simulink model "PrkgClimaActvMgr"  (R2022b) (continued)
== system PrkgClimaActvMgr/PrkgClimaActvMgr_Runnable_sys == (continued)
      settings: EnsureOutportIsVirtual=on
  PrkgClimaOnReq_PrkgClimaOnReq_write : Outport  Port=116
      settings: EnsureOutportIsVirtual=on
  PrkgClimaPostCdngReq_PrkgClimaPostCdngReq_write : Outport  Port=117
      settings: EnsureOutportIsVirtual=on
  PrkgClimaPreCdngReq_PrkgClimaPreCdngReq_write : Outport  Port=118
      settings: EnsureOutportIsVirtual=on
  PrkgClimaRecirReq_PrkgClimaRecirReq_write : Outport  Port=119
      settings: EnsureOutportIsVirtual=on
  PrkgClimaRqrdImdtForBattULo_PrkgClimaRqrdImdtForBattULo_write : Outport  Port=120
      settings: EnsureOutportIsVirtual=on
  PrkgClimaRqrdImdtFromActvMgr_PrkgClimaRqrdImdtFromActvMgr_write : Outport  Port=121
      settings: EnsureOutportIsVirtual=on
  PrkgClimaRqrdTmrForBattULo_PrkgClimaRqrdTmrForBattULo_write : Outport  Port=122
      settings: EnsureOutportIsVirtual=on
  PrkgClimaTemp_PrkgClimaTemp_write : Outport  Port=123
      settings: EnsureOutportIsVirtual=on
  PrkgClimaWarn_PrkgClimaWarn_write : Outport  Port=124
      settings: EnsureOutportIsVirtual=on
  PrkgModMgr_PrkgModMgr_write : Outport  Port=125
      settings: EnsureOutportIsVirtual=on
  RemClimaActv_RemClimaActv_write : Outport  Port=126
      settings: EnsureOutportIsVirtual=on
  RemClimaDefrstSts_RemClimaDefrstSts_write : Outport  Port=127
      settings: EnsureOutportIsVirtual=on
  RemClimaDelaySts_RemClimaDelaySts_write : Outport  Port=128
      settings: EnsureOutportIsVirtual=on
  RemClimaEgyFinalReq_RemClimaEgyFinalReq_write : Outport  Port=129
      settings: EnsureOutportIsVirtual=on
  RemClimaExtnTiRspn_RemClimaExtnTiRspn_write : Outport  Port=130
      settings: EnsureOutportIsVirtual=on
  RemClimaFnshd_RemClimaFnshd_write : Outport  Port=131
      settings: EnsureOutportIsVirtual=on
  RemClimaHvSts_RemClimaHvSts_write : Outport  Port=132
      settings: EnsureOutportIsVirtual=on
  RemStrtClimaRspn_RemStrtClimaRspn_write : Outport  Port=133
      settings: EnsureOutportIsVirtual=on
  RemVentActvSts_RemVentActvSts_write : Outport  Port=134
      settings: EnsureOutportIsVirtual=on
  RemVentFbSts_RemVentFbSts_write : Outport  Port=135
      settings: EnsureOutportIsVirtual=on
  RemVentReqRspnFb_RemVentReqRspnFb_write : Outport  Port=136
      settings: EnsureOutportIsVirtual=on
  RemVentWarnSts_RemVentWarnSts_write : Outport  Port=137
      settings: EnsureOutportIsVirtual=on
  ReqForPrkgClima_ReqForPrkgClima_write : Outport  Port=138
      settings: EnsureOutportIsVirtual=on
  ReqForSwtHeatrTypFromActvMgr_ReqForSwtHeatrTypFromActvMgr_write : Outport  Port=139
      settings: EnsureOutportIsVirtual=on
  RoofOpnReqFrmClima_RoofOpnReqFrmClima_write : Outport  Port=140
      settings: EnsureOutportIsVirtual=on
  RtLeForPrkgClima_RtLeForPrkgClima_write : Outport  Port=141
      settings: EnsureOutportIsVirtual=on
  SchedCmptHeatCoolFB_SchedCmptHeatCoolFB_write : Outport  Port=142
      settings: EnsureOutportIsVirtual=on
  SeatClimaDurgClima_SeatClimaDurgClima_write : Outport  Port=143
      settings: EnsureOutportIsVirtual=on
  SteerWhlHeatgDurgClima_Ngcc_SteerWhlHeatgDurgClima_write : Outport  Port=144
      settings: EnsureOutportIsVirtual=on
  SuspStrtActvForPrkgClima_SuspStrtActvForPrkgClima_write : Outport  Port=145
      settings: EnsureOutportIsVirtual=on
  WarnForHybWoUFromActvMgr_WarnForHybWoUFromActvMgr_write : Outport  Port=146
      settings: EnsureOutportIsVirtual=on
  wires:
  ActvRqrdForPostPrkgClima_ActvRqrdForPostPrkgClima_read/1 -> Terminator_1/1
  CarTiIntForClima_CarTiIntForClima_read/1 -> Terminator_2/1
  CdsClimaOnReqfromExsrc_CdsClimaOnReqfromExsrc_read/1 -> Terminator_3/1
  ClimaCfgForClimaCtrlMan1_ClimaCfgForClimaCtrlMan1_read/1 -> Terminator_4/1
  ClimaCfgForCmptmtClng_ClimaCfgForCmptmtClng_read/1 -> Terminator_5/1
  ClimaCfgForFoldCSD_ClimaCfgForFoldCSD_read/1 -> Terminator_6/1
  ClimaCfgForNrOfTSetg1_ClimaCfgForNrOfTSetg1_read/1 -> Terminator_7/1
  ClimaCfgForPreClng1_ClimaCfgForPreClng1_read/1 -> Terminator_8/1
  ClimaCfgForSeatHeatgForRowFirstPrsnt1_ClimaCfgForSeatHeatgForRowFirstPrsnt1_read/1 -> Terminator_9/1
  ClimaCfgForSeatHeatgForRowSec_ClimaCfgForSeatHeatgForRowSec_read/1 -> Terminator_10/1
  ClimaCfgForSeatVentnForRowFirst_ClimaCfgForSeatVentnForRowFirst_read/1 -> Terminator_11/1
  ClimaCfgForSeatVentnForRowSec_ClimaCfgForSeatVentnForRowSec_read/1 -> Terminator_12/1
  ClimaCfgForSteerWhlHeatgPrsnt1_ClimaCfgForSteerWhlHeatgPrsnt1_read/1 -> Terminator_13/1
  ClimaCfgForSteerWhlLeSide1_ClimaCfgForSteerWhlLeSide1_read/1 -> Terminator_14/1
  ClimaCfgForVehElec1_ClimaCfgForVehElec1_read/1 -> Terminator_15/1
  ClimaCfgForVehHyb1_ClimaCfgForVehHyb1_read/1 -> Terminator_16/1
  ClimaCfgForWindHeatrPrsnt1_ClimaCfgForWindHeatrPrsnt1_read/1 -> Terminator_17/1
  ClimaOvrHeatProWarnIni_ClimaOvrHeatProWarnIni_read/1 -> Terminator_18/1
  ClimaOvrHeatReqFromExrsc_ClimaOvrHeatReqFromExrsc_read/1 -> Terminator_19/1
  ClimaOvrHeatStrtReq_ClimaOvrHeatStrtReq_read/1 -> Terminator_20/1
  CmptmtClsd_CmptmtClsd_read/1 -> Terminator_21/1
  CSDUsgChgeOffReq_CSDUsgChgeOffReq_read/1 -> Terminator_22/1
  CSDUsgChgeOnReq_CSDUsgChgeOnReq_read/1 -> Terminator_23/1
  DefrstHeatgForPrkgClimaEnad_DefrstHeatgForPrkgClimaEnad_read/1 -> Terminator_24/1
  DoorOpenStsForClima_DoorOpenStsForClima_read/1 -> Terminator_25/1
  EgyAllwdForPrkgClima_EgyAllwdForPrkgClima_read/1 -> Terminator_26/1
  EgyPrioForPrkgClima_EgyPrioForPrkgClima_read/1 -> Terminator_27/1
  ErsCmdByKeyStrtReq_ErsCmdByKeyStrtReq_read/1 -> Terminator_28/1
  ErsRunTimelStrtReq_ErsRunTimelStrtReq_read/1 -> Terminator_29/1
  FacForRtForPrkgClima_FacForRtForPrkgClima_read/1 -> Terminator_30/1
  FrntHvacBlowerSts_FrntHvacBlowerSts_read/1 -> Terminator_31/1
  HeatrTypForPrkgClima_HeatrTypForPrkgClima_read/1 -> Terminator_32/1
  HeatUpRqrdForPrkgClima_HeatUpRqrdForPrkgClima_read/1 -> Terminator_33/1
  HMIExtVentFanVolReqStrtReq_HMIExtVentFanVolReqStrtReq_read/1 -> Terminator_34/1
  HMIExtVentonoffReqStrtReq_HMIExtVentonoffReqStrtReq_read/1 -> Terminator_35/1
  HMIExtVentTSpReqStrtReq_HMIExtVentTSpReqStrtReq_read/1 -> Terminator_36/1
  HMIExtVentTStsReqStrtReq_HMIExtVentTStsReqStrtReq_read/1 -> Terminator_37/1
  HvBattForPrkgClima_HvBattForPrkgClima_read/1 -> Terminator_38/1
  HvBattSoc_HvBattSoc_read/1 -> Terminator_39/1
  HvSysCnctWoU_HvSysCnctWoU_read/1 -> Terminator_40/1
  HvSysWoU_HvSysWoU_read/1 -> Terminator_41/1
  HvSysRlySts_HvSysRlySts_read/1 -> Terminator_42/1
  IntCo2MontrStrtReq_IntCo2MontrStrtReq_read/1 -> Terminator_43/1
  LockgCenSts_Ngcc_LockgCenSts_read/1 -> Terminator_44/1
  MemReadForPrkgClimaActvMgr_MemReadForPrkgClimaActvMgr_read/1 -> Terminator_45/1
  MemReqStsForPrkgClima_MemReqStsForPrkgClima_read/1 -> Terminator_46/1
  OffForTLoForPostCdng_OffForTLoForPostCdng_read/1 -> Terminator_47/1
  OffRqrdForPrkgClima_OffRqrdForPrkgClima_read/1 -> Terminator_48/1
  PrePrkgClimaNotAvlForHvSysWoU_PrePrkgClimaNotAvlForHvSysWoU_read/1 -> Terminator_49/1
  PrkgCDSAvl_PrkgCDSAvl_read/1 -> Terminator_50/1
  PrkgClimaAllwdForBattULo_PrkgClimaAllwdForBattULo_read/1 -> Terminator_51/1
  PrkgClimaAllwdForSwtHeatrTyp_PrkgClimaAllwdForSwtHeatrTyp_read/1 -> Terminator_52/1
  PrkgHeatgActv_PrkgHeatgActv_read/1 -> Terminator_53/1
  PwrAllwdForHvacFanFrnt_PwrAllwdForHvacFanFrnt_read/1 -> Terminator_54/1
  PwrCnsDesForHvacFanFrnt_PwrCnsDesForHvacFanFrnt_read/1 -> Terminator_55/1
  RemClimaallExsrcReq_RemClimaallExsrcReq_read/1 -> Terminator_56/1
  RemClimaEgyExsrcReq_RemClimaEgyExsrcReq_read/1 -> Terminator_57/1
  RemClimaTmrStrtReq_RemClimaTmrStrtReq_read/1 -> Terminator_58/1
  RemStrtExtnTiStrtReq_RemStrtExtnTiStrtReq_read/1 -> Terminator_59/1
  RemStrtHvCtrlStrtReq_RemStrtHvCtrlStrtReq_read/1 -> Terminator_60/1
  RemVentReqStrtReq_RemVentReqStrtReq_read/1 -> Terminator_61/1
  RtCmftForImdtPrePrkgClima_RtCmftForImdtPrePrkgClima_read/1 -> Terminator_62/1
  RtCmftForPostPrkgClima_RtCmftForPostPrkgClima_read/1 -> Terminator_63/1
  RtCmftForPreClng_RtCmftForPreClng_read/1 -> Terminator_64/1
  RtSdlForPrkgClima_RtSdlForPrkgClima_read/1 -> Terminator_65/1
  SeatHeatForPrkgClimaEnad_SeatHeatForPrkgClimaEnad_read/1 -> Terminator_66/1
  SteerWhlHeatgForPrkgClimaEnad_SteerWhlHeatgForPrkgClimaEnad_read/1 -> Terminator_67/1
  StrtRemSts1WdStrtRemSts_Ngcc_StrtRemSts1WdStrtRemSts_read/1 -> Terminator_68/1
  StrtRqrdForDirForPrePrkgClima_StrtRqrdForDirForPrePrkgClima_read/1 -> Terminator_69/1
  StrtRqrdForPreClng_StrtRqrdForPreClng_read/1 -> Terminator_70/1
  StrtRqrdForTmrForPrePrkgClima_StrtRqrdForTmrForPrePrkgClima_read/1 -> Terminator_71/1
  SuspStrtAllwdForPrkgClima_SuspStrtAllwdForPrkgClima_read/1 -> Terminator_72/1
  TelmBookClimaReqHmiCmptmtTSpSpclStrtReq_TelmBookClimaReqHmiCmptmtTSpSpclStrtReq_read/1 -> Terminator_73/1
  TelmBookClimaReqOnOffNoReqStrtReq_TelmBookClimaReqOnOffNoReqStrtReq_read/1 -> Terminator_74/1
  TelmBookClimaReqTempRangeStrtReq_TelmBookClimaReqTempRangeStrtReq_read/1 -> Terminator_75/1
  TelmBookClimaTmrStrtReq_TelmBookClimaTmrStrtReq_read/1 -> Terminator_76/1
  TelmBookSeatHeatClimalReqDrvHeatClimaLvlStrtReq_TelmBookSeatHeatClimalReqDrvHeatClimaLvlStrtReq_read/1 -> Terminator_77/1
  TelmBookSeatHeatClimalReqPassHeatClimaLvlStrtReq_TelmBookSeatHeatClimalReqPassHeatClimaLvlStrtReq_read/1 -> Terminator_78/1
  TelmBookSeatHeatClimalReqSecLeHeatClimaLvlStrtReq_TelmBookSeatHeatClimalReqSecLeHeatClimaLvlStrtReq_read/1 -> Terminator_79/1
  TelmBookSeatHeatClimalReqSecRiHeatClimaLvlStrtReq_TelmBookSeatHeatClimalReqSecRiHeatClimaLvlStrtReq_read/1 -> Terminator_80/1
  TelmBookSeatHeatClimalReqThrdLeHeatClimaLvlStrtReq_TelmBookSeatHeatClimalReqThrdLeHeatClimaLvlStrtReq_read/1 -> Terminator_81/1
  TelmBookSeatHeatClimalReqThrdRiHeatClimaLvlStrtReq_TelmBookSeatHeatClimalReqThrdRiHeatClimaLvlStrtReq_read/1 -> Terminator_82/1
  TelmBookSeatVentnClimalReqDrvVentnClimaLvlStrtReq_TelmBookSeatVentnClimalReqDrvVentnClimaLvlStrtReq_read/1 -> Terminator_83/1
  TelmBookSeatVentnClimalReqPassVentnClimaLvlStrtReq_TelmBookSeatVentnClimalReqPassVentnClimaLvlStrtReq_read/1 -> Terminator_84/1
  TelmBookSeatVentnClimalReqSecLeVentnClimaLvlStrtReq_TelmBookSeatVentnClimalReqSecLeVentnClimaLvlStrtReq_read/1 -> Terminator_85/1
  TelmBookSeatVentnClimalReqSecRiVentnClimaLvlStrtReq_TelmBookSeatVentnClimalReqSecRiVentnClimaLvlStrtReq_read/1 -> Terminator_86/1
  TelmBookSeatVentnClimalReqThrdLeVentnClimaLvlStrtReq_TelmBookSeatVentnClimalReqThrdLeVentnClimaLvlStrtReq_read/1 -> Terminator_87/1
  TelmBookSeatVentnClimalReqThrdRiVentnClimaLvlStrtReq_TelmBookSeatVentnClimalReqThrdRiVentnClimaLvlStrtReq_read/1 -> Terminator_88/1
  TelmBookSteerWhlHeatClimalReqStrtReq_TelmBookSteerWhlHeatClimalReqStrtReq_read/1 -> Terminator_89/1
  TelmClimaExtVentnLvlStrtReq_TelmClimaExtVentnLvlStrtReq_read/1 -> Terminator_90/1
  TelmClimaExtVentnReqStrtReq_TelmClimaExtVentnReqStrtReq_read/1 -> Terminator_91/1
  TelmClimaExtVentnTempRangeStrtReq_TelmClimaExtVentnTempRangeStrtReq_read/1 -> Terminator_92/1
  TelmClimaExtVentnTSpSpclStrtReq_TelmClimaExtVentnTSpSpclStrtReq_read/1 -> Terminator_93/1
  UpdRtTotForTmrForPrePrkgClima_UpdRtTotForTmrForPrePrkgClima_read/1 -> Terminator_94/1
  VehModMngtGlbSafe1_Ngcc_VehModMngtGlbSafe1_read/1 -> Terminator_95/1
  VFCBodyPreClimatisationHmiHumCtrl_VFCBodyPreClimatisationHmiHumCtrl_read/1 -> Terminator_96/1
  VFCHmiClimaCtrlAct_VFCHmiClimaCtrlAct_read/1 -> Terminator_97/1
  VFCPrkDrvgClimabyFragAct_VFCPrkDrvgClimabyFragAct_read/1 -> Terminator_98/1
  WarnForEgyAllwdLimd_WarnForEgyAllwdLimd_read/1 -> Terminator_99/1
  WarnForHybWoU_WarnForHybWoU_read/1 -> Terminator_100/1
  WarnForPreCdngFctNotAvl_WarnForPreCdngFctNotAvl_read/1 -> Terminator_101/1
  WarnForPrkgClimaNotAllwd_WarnForPrkgClimaNotAllwd_read/1 -> Terminator_102/1
  WindIceDetdFrnt_Ngcc_WindIceDetdFrnt_read/1 -> Terminator_103/1
  WindIceDetdRe_Ngcc_WindIceDetdRe_read/1 -> Terminator_104/1
  AirCdnAvl_AirCdnAvl_read/1 -> Terminator_105/1
  AmbTFildForClimaNgcc_AmbTFildForClima_read/1 -> Terminator_106/1
  OffRqrdForRemClima_OffRqrdForRemClima_read/1 -> Terminator_107/1
  RemClimaTempStrtReq_RemClimaTempStrtReq_read/1 -> Terminator_108/1
  VFCAutDemstOperSts_VFCAutDemstOperSts_read/1 -> Terminator_109/1
  VFCPrkDrvgClimabyPM25_VFCPrkDrvgClimabyPM25_read/1 -> Terminator_110/1
  AirCdnAvlRe_AirCdnAvlRe_read/1 -> Terminator_111/1
  AirSrcStsFbNgcc_AirSrcStsFbNgcc_read/1 -> Terminator_112/1
  BookTrvlClimaActvReqfromExsrc_BookTrvlClimaActvReqfromExsrc_read/1 -> Terminator_113/1
  CCMWakeupFunction_CCMWakeupFunction_read/1 -> Terminator_114/1
  ClimaCfgFor3RDROWSEATHEAT_ClimaCfgFor3RDROWSEATHEAT_read/1 -> Terminator_115/1
  ClimaCfgFor3RDROWSEATVENTILATED_ClimaCfgFor3RDROWSEATVENTILATED_read/1 -> Terminator_116/1
  ClimaCfgForAirMtrlSnsrPrsnt1_ClimaCfgForAirMtrlSnsrPrsnt1_read/1 -> Terminator_117/1
  ClimaCfgForBRAND_ClimaCfgForBRAND_read/1 -> Terminator_118/1
  ClimaCfgForDrvrSeatVentWthSmf2_ClimaCfgForDrvrSeatVentWthSmf2_read/1 -> Terminator_119/1
  ClimaCfgForErev1_ClimaCfgForErev1_read/1 -> Terminator_120/1
  ClimaCfgForHvacRePrsnt1_ClimaCfgForHvacRePrsnt1_read/1 -> Terminator_121/1
  ClimaCfgForHvacTyp_ClimaCfgForHvacTyp_read/1 -> Terminator_122/1
  ClimaCfgForLifeDetection_ClimaCfgForLifeDetection_read/1 -> Terminator_123/1
  ClimaCfgForMARKET_ClimaCfgForMARKET_read/1 -> Terminator_124/1
  ClimaCfgForPassSeatVentWthSmf2_ClimaCfgForPassSeatVentWthSmf2_read/1 -> Terminator_125/1
  ClimaCfgForPrpsnTyp_ClimaCfgForPrpsnTyp_read/1 -> Terminator_126/1
  ClimaCfgForRoofHatch_ClimaCfgForRoofHatch_read/1 -> Terminator_127/1
  ClimaCfgForVehTyp_ClimaCfgForVehTyp_read/1 -> Terminator_128/1
  ClimaCfgReqVersionReplace_ClimaCfgReqVersionReplace_read/1 -> Terminator_129/1
  CmptmtClngActv_CmptmtClngActv_read/1 -> Terminator_130/1
  CmptmtOpenStsForClima_CmptmtOpenStsForClima_read/1 -> Terminator_131/1
  DoorStsWithOutTrForClima_DoorStsWithOutTrForClima_read/1 -> Terminator_132/1
  DrvrSeatHeatgLvlSts_DrvrSeatHeatgLvlSts_read/1 -> Terminator_133/1
  DrvrSeatHeatgLvlSts_DrvrSeatHeatgLvlSts_IsUpdated_read/1 -> Terminator_134/1
  DrvrSeatVentnLvlSts_DrvrSeatVentnLvlSts_read/1 -> Terminator_135/1
  DrvrSeatVentnLvlSts_DrvrSeatVentnLvlSts_IsUpdated_read/1 -> Terminator_136/1
  DrvrSeatVentnLvlSts2Ngcc_DrvrSeatVentnLvlSts2Ngcc_read/1 -> Terminator_137/1
  EngSt1WdSts_Ngcc_EngSt1WdSts_read/1 -> Terminator_138/1
  ErsCmdByKeyFromExsrc_ErsCmdByKeyFromExsrc_read/1 -> Terminator_139/1
  GearLvrIndcn_GearLvrIndcn_read/1 -> Terminator_140/1
  GearLvrIndcn_GearLvrIndcn_IsUpdated_read/1 -> Terminator_141/1
  HighTempCleanHmiReqNgcc_HighTempCleanHmiReqNgcc_read/1 -> Terminator_142/1
  HMIAirCdnrAutDrySetNgcc_HMIAirCdnrAutDrySetNgcc_read/1 -> Terminator_143/1
  HMIAirCdnrAutDrySetNgcc_HMIAirCdnrAutDrySetNgcc_IsUpdated_read/1 -> Terminator_144/1
  HmiCmptmtTSp_HmiCmptmtTSp_read/1 -> Terminator_145/1
  HMILBRACStrtSetNgcc_HMILBRACStrtSetNgcc_read/1 -> Terminator_146/1
  HmiParkVentSetNgcc_HmiParkVentSetNgcc_read/1 -> Terminator_147/1
  HmiParkVentTmrSetNgcc_HmiParkVentTmrSetNgcc_read/1 -> Terminator_148/1
  HvBattMntnActv_HvBattMntnActv_read/1 -> Terminator_149/1
  HvCooltHeatrStsSig_HvCooltHeatrStsSig_read/1 -> Terminator_150/1
  HvCooltHeatrStsSig_HvCooltHeatrStsSig_IsUpdated_read/1 -> Terminator_151/1
  IntPm25VluFrmClima_IntPm25VluFrmClima_read/1 -> Terminator_152/1
  LDACState_LDACState_read/1 -> Terminator_153/1
  MemBookTrvlClimaTi_MemBookTrvlClimaTi_read/1 -> Terminator_154/1
  MemReadForBlwAftRun_MemReadForBlwAftRun_read/1 -> Terminator_155/1
  MemReadForHighTempCln_MemReadForHighTempCln_read/1 -> Terminator_156/1
  MemReadForHMISetEngReq_MemReadForHMISetEngReq_read/1 -> Terminator_157/1
  MemReadForParkVent_MemReadForParkVent_read/1 -> Terminator_158/1
  MemReadForParkVentActvCnt_MemReadForParkVentActvCnt_read/1 -> Terminator_159/1
  OnBdChrgrSt_OnBdChrgrSt_read/1 -> Terminator_160/1
  OnBdChrgrSt_OnBdChrgrSt_IsUpdated_read/1 -> Terminator_161/1
  OxygenSplyAvlStsNgcc_OxygenSplyAvlStsNgcc_read/1 -> Terminator_162/1
  OxygenSplyErrNgcc_OxygenSplyErrNgcc_read/1 -> Terminator_163/1
  PassSeatHeatgLvlSts_PassSeatHeatgLvlSts_read/1 -> Terminator_164/1
  PassSeatHeatgLvlSts_PassSeatHeatgLvlSts_IsUpdated_read/1 -> Terminator_165/1
  PassSeatVentnLvlSts_PassSeatVentnLvlSts_read/1 -> Terminator_166/1
  PassSeatVentnLvlSts_PassSeatVentnLvlSts_IsUpdated_read/1 -> Terminator_167/1
  PassSeatVentnLvlSts2Ngcc_PassSeatVentnLvlSts2Ngcc_read/1 -> Terminator_168/1
  PrkgClimaReqExcpSeat_PrkgClimaReqExcpSeat_read/1 -> Terminator_169/1
  PrkgClimaReqPrio_PrkgClimaReqPrio_read/1 -> Terminator_170/1
  PrkgOGMAirSrcReq_PrkgOGMAirSrcReq_read/1 -> Terminator_171/1
  PrvntWinActvReq_PrvntWinActvReq_read/1 -> Terminator_172/1
  ReHvacBlowerSts_ReHvacBlowerSts_read/1 -> Terminator_173/1
  RemClimaEgyExsrcReqPlus_RemClimaEgyExsrcReqPlus_read/1 -> Terminator_174/1
  RemClimaHVReqFb_RemClimaHVReqFb_read/1 -> Terminator_175/1
  RemClimaHVReqFb_RemClimaHVReqFb_IsUpdated_read/1 -> Terminator_176/1
  RemClimaWarnForCmptmtClng_RemClimaWarnForCmptmtClng_read/1 -> Terminator_177/1
  RemClimaWarnForOpenGlb_RemClimaWarnForOpenGlb_read/1 -> Terminator_178/1
  RemClimaWarnForUnLckClima_RemClimaWarnForUnLckClima_read/1 -> Terminator_179/1
  RemOxygenSplyReq_RemOxygenSplyReq_read/1 -> Terminator_180/1
  SeatHeatgLvlStsRowSecLe_SeatHeatgLvlStsRowSecLe_read/1 -> Terminator_181/1
  SeatHeatgLvlStsRowSecLe_SeatHeatgLvlStsRowSecLe_IsUpdated_read/1 -> Terminator_182/1
  SeatHeatgLvlStsRowSecRi_SeatHeatgLvlStsRowSecRi_read/1 -> Terminator_183/1
  SeatHeatgLvlStsRowSecRi_SeatHeatgLvlStsRowSecRi_IsUpdated_read/1 -> Terminator_184/1
  SeatHeatgLvlStsRowThrdLe_SeatHeatgLvlStsRowThrdLe_read/1 -> Terminator_185/1
  SeatHeatgLvlStsRowThrdLe_SeatHeatgLvlStsRowThrdLe_IsUpdated_read/1 -> Terminator_186/1
  SeatHeatgLvlStsRowThrdRi_SeatHeatgLvlStsRowThrdRi_read/1 -> Terminator_187/1
  SeatHeatgLvlStsRowThrdRi_SeatHeatgLvlStsRowThrdRi_IsUpdated_read/1 -> Terminator_188/1
  SeatVentnLvlStsRowSecLe_SeatVentnLvlStsRowSecLe_read/1 -> Terminator_189/1
  SeatVentnLvlStsRowSecLe_SeatVentnLvlStsRowSecLe_IsUpdated_read/1 -> Terminator_190/1
  SeatVentnLvlStsRowSecRi_SeatVentnLvlStsRowSecRi_read/1 -> Terminator_191/1
  SeatVentnLvlStsRowSecRi_SeatVentnLvlStsRowSecRi_IsUpdated_read/1 -> Terminator_192/1
  SeatVentnLvlStsRowThrdLe_SeatVentnLvlStsRowThrdLe_read/1 -> Terminator_193/1
  SeatVentnLvlStsRowThrdLe_SeatVentnLvlStsRowThrdLe_IsUpdated_read/1 -> Terminator_194/1
  SeatVentnLvlStsRowThrdRi_SeatVentnLvlStsRowThrdRi_read/1 -> Terminator_195/1
  SeatVentnLvlStsRowThrdRi_SeatVentnLvlStsRowThrdRi_IsUpdated_read/1 -> Terminator_196/1
  SteerWhlHeatgDurgClimaEnadFromExsrc_SteerWhlHeatgDurgClimaEnadFromExsrc_read/1 -> Terminator_197/1
  SunRoofPosnSts_Ngcc_SunRoofPosnSts_read/1 -> Terminator_198/1
  TcsOvrheatdProtnReq_TcsOvrheatdProtnReq_read/1 -> Terminator_199/1
  TelmClimaTmrFromExtSrc_TelmClimaTmrFromExtSrc_read/1 -> Terminator_200/1
  TelmDefrostTmr_TelmDefrostTmr_read/1 -> Terminator_201/1
  TelmDefrostTmr_TelmDefrostTmr_IsUpdated_read/1 -> Terminator_202/1
  TelmOxygenReqFromExSrc_TelmOxygenReqFromExSrc_read/1 -> Terminator_203/1
  TelmOxygenTmr_TelmOxygenTmr_read/1 -> Terminator_204/1
  TelmOxygenTmr_TelmOxygenTmr_IsUpdated_read/1 -> Terminator_205/1
  TelmSeatDrvrHeatReqFromExsrc_TelmSeatDrvrHeatReqFromExsrc_read/1 -> Terminator_206/1
  TelmSeatDrvVentnReqFromExsrc_TelmSeatDrvVentnReqFromExsrc_read/1 -> Terminator_207/1
  TelmSeatHeatDrvrTmr_TelmSeatHeatDrvrTmr_read/1 -> Terminator_208/1
  TelmSeatHeatDrvrTmr_TelmSeatHeatDrvrTmr_IsUpdated_read/1 -> Terminator_209/1
  TelmSeatHeatPassTmr_TelmSeatHeatPassTmr_read/1 -> Terminator_210/1
  TelmSeatHeatPassTmr_TelmSeatHeatPassTmr_IsUpdated_read/1 -> Terminator_211/1
  TelmSeatHeatSecLeTmr_TelmSeatHeatSecLeTmr_read/1 -> Terminator_212/1
  TelmSeatHeatSecLeTmr_TelmSeatHeatSecLeTmr_IsUpdated_read/1 -> Terminator_213/1
  TelmSeatHeatSecRiTmr_TelmSeatHeatSecRiTmr_read/1 -> Terminator_214/1
  TelmSeatHeatSecRiTmr_TelmSeatHeatSecRiTmr_IsUpdated_read/1 -> Terminator_215/1
  TelmSeatHeatThrdLeTmr_TelmSeatHeatThrdLeTmr_read/1 -> Terminator_216/1
  TelmSeatHeatThrdLeTmr_TelmSeatHeatThrdLeTmr_IsUpdated_read/1 -> Terminator_217/1
  TelmSeatHeatThrdRiTmr_TelmSeatHeatThrdRiTmr_read/1 -> Terminator_218/1
  TelmSeatHeatThrdRiTmr_TelmSeatHeatThrdRiTmr_IsUpdated_read/1 -> Terminator_219/1
  TelmSeatPassHeatReqFromExsrc_TelmSeatPassHeatReqFromExsrc_read/1 -> Terminator_220/1
  TelmSeatPassVentnReqFromExsrc_TelmSeatPassVentnReqFromExsrc_read/1 -> Terminator_221/1
  TelmSeatSecLeHeatReqFromExsrc_TelmSeatSecLeHeatReqFromExsrc_read/1 -> Terminator_222/1
  TelmSeatSecLeVentnReqFromExsrc_TelmSeatSecLeVentnReqFromExsrc_read/1 -> Terminator_223/1
  TelmSeatSecRiHeatReqFromExsrc_TelmSeatSecRiHeatReqFromExsrc_read/1 -> Terminator_224/1
  TelmSeatSecRiVentnReqFromExsrc_TelmSeatSecRiVentnReqFromExsrc_read/1 -> Terminator_225/1
  TelmSeatThrdLeHeatReqFromExsrc_TelmSeatThrdLeHeatReqFromExsrc_read/1 -> Terminator_226/1
  TelmSeatThrdLeVentnReqFromExsrc_TelmSeatThrdLeVentnReqFromExsrc_read/1 -> Terminator_227/1
  TelmSeatThrdRiHeatReqFromExsrc_TelmSeatThrdRiHeatReqFromExsrc_read/1 -> Terminator_228/1
  TelmSeatThrdRiVentnReqFromExsrc_TelmSeatThrdRiVentnReqFromExsrc_read/1 -> Terminator_229/1
  TelmSeatVentnDrvrTmr_TelmSeatVentnDrvrTmr_read/1 -> Terminator_230/1
  TelmSeatVentnDrvrTmr_TelmSeatVentnDrvrTmr_IsUpdated_read/1 -> Terminator_231/1
  TelmSeatVentnPassTmr_TelmSeatVentnPassTmr_read/1 -> Terminator_232/1
  TelmSeatVentnPassTmr_TelmSeatVentnPassTmr_IsUpdated_read/1 -> Terminator_233/1
  TelmSeatVentnSecLeTmr_TelmSeatVentnSecLeTmr_read/1 -> Terminator_234/1
  TelmSeatVentnSecLeTmr_TelmSeatVentnSecLeTmr_IsUpdated_read/1 -> Terminator_235/1
  TelmSeatVentnSecRiTmr_TelmSeatVentnSecRiTmr_read/1 -> Terminator_236/1
  TelmSeatVentnSecRiTmr_TelmSeatVentnSecRiTmr_IsUpdated_read/1 -> Terminator_237/1
  TelmSeatVentnThrdLeTmr_TelmSeatVentnThrdLeTmr_read/1 -> Terminator_238/1
  TelmSeatVentnThrdLeTmr_TelmSeatVentnThrdLeTmr_IsUpdated_read/1 -> Terminator_239/1
  TelmSeatVentnThrdReq_TelmSeatVentnThrdReq_read/1 -> Terminator_240/1
  TelmSeatVentnThrdRiTmr_TelmSeatVentnThrdRiTmr_read/1 -> Terminator_241/1
  TelmSeatVentnThrdRiTmr_TelmSeatVentnThrdRiTmr_IsUpdated_read/1 -> Terminator_242/1
  TelmSteerWhlHeatTmr_TelmSteerWhlHeatTmr_read/1 -> Terminator_243/1
  TelmSteerWhlHeatTmr_TelmSteerWhlHeatTmr_IsUpdated_read/1 -> Terminator_244/1
  UnLckClimaActvReq_UnLckClimaActvReq_read/1 -> Terminator_245/1
  UnLckClimaActvReqSt_UnLckClimaActvReqSt_read/1 -> Terminator_246/1
  UnLckHeatActvReq_UnLckHeatActvReq_read/1 -> Terminator_247/1
  UnLckHeatActvReqSt_UnLckHeatActvReqSt_read/1 -> Terminator_248/1
  VentnForWinOpenGlbActv_VentnForWinOpenGlbActv_read/1 -> Terminator_249/1
  VFCPrkDrvgClimabyOxygenSplyCtrl_VFCPrkDrvgClimabyOxygenSplyCtrl_read/1 -> Terminator_250/1
  WarnLvlMsgNgcc_WarnLvlMsgNgcc_read/1 -> Terminator_251/1
  WarnLvlMsgNgcc_WarnLvlMsgNgcc_IsUpdated_read/1 -> Terminator_252/1
  WinOpenGlbReq_WinOpenGlbReq_read/1 -> Terminator_253/1
  WinPosnStsAtReLe_WinPosnStsAtReLe_read/1 -> Terminator_254/1
  WinPosnStsAtReRi_WinPosnStsAtReRi_read/1 -> Terminator_255/1
  WinPosnStsDrv_WinPosnStsAtDrv_read/1 -> Terminator_256/1
  WinPosnStsPass_WinPosnStsAtPass_read/1 -> Terminator_257/1
  Ground_1/1 -> BlwAftRunAct_BlwAftRunAct_write/1
  Ground_2/1 -> BlwAftRunReqRe_BlwAftRunReqRe_write/1
  Ground_3/1 -> BlwAftRunWkTime_BlwAftRunWkTime_write/1
  Ground_4/1 -> BookTrvlClimaFnshd_BookTrvlClimaFnshd_write/1
  Ground_5/1 -> DsChrgClima_DsChrgClima_write/1
  Ground_6/1 -> HghClnAct_HghClnAct_write/1
  Ground_7/1 -> HghClnWkTime_HghClnWkTime_write/1
  Ground_8/1 -> HighTempCleanRunningStsNgcc_HighTempCleanRunningStsNgcc_write/1
  Ground_9/1 -> HighTempCleanSettingStsNgcc_HighTempCleanSettingStsNgcc_write/1
  Ground_10/1 -> HighTempClnActv_HighTempClnActv_write/1
  Ground_11/1 -> KeyClimaActvFnshd_KeyClimaActvFnshd_write/1
  Ground_12/1(Out1) -> KeySynchrnztnLclNgcc_KeySynchrnztnLclNgcc_write/1
  Ground_13/1 -> MemSaveFlgForBlwAftRun_MemSaveFlgForBlwAftRun_write/1
  Ground_14/1 -> MemSaveFlgForHighTempCln_MemSaveFlgForHighTempCln_write/1
  Ground_15/1 -> MemSaveFlgForHMISetEngReq_MemSaveFlgForHMISetEngReq_write/1
  Ground_16/1 -> MemSaveFlgForParkVent_MemSaveFlgForParkVent_write/1
  Ground_17/1 -> MemSaveFlgForParkVentActvCnt_MemSaveFlgForParkVentActvCnt_write/1
  Ground_18/1(Out1) -> MemWrForBlwAftRun_MemWrForBlwAftRun_write/1
  Ground_19/1(Out1) -> MemWrForHighTempCln_MemWrForHighTempCln_write/1
  Ground_20/1(Out1) -> MemWrForHMISetEngReq_MemWrForHMISetEngReq_write/1
  Ground_21/1(Out1) -> MemWrForParkVent_MemWrForParkVent_write/1
  Ground_22/1(Out1) -> MemWrForParkVentActvCnt_MemWrForParkVentActvCnt_write/1
  Ground_23/1 -> OGMAirSrcFnshd_OGMAirSrcFnshd_write/1
  Ground_24/1 -> OGMAirSrcReq_OGMAirSrcReq_write/1
  Ground_25/1 -> OGMAvlSts_OGMAvlSts_write/1
  Ground_26/1 -> OGMReq_OGMReq_write/1
  Ground_27/1 -> ParkVentActv_ParkVentActv_write/1
  Ground_28/1 -> ParkVentWkTi_ParkVentWkTi_write/1
  Ground_29/1 -> PrkgClimaACReq_PrkgClimaACReq_write/1
  Ground_30/1 -> PrkgClimaActvMgrVFCLDACClimaCtrl_PrkgClimaActvMgrVFCLDACClimaCtrl_write/1
  Ground_31/1 -> PrkgClimaAirflowReqRe_PrkgClimaAirflowReqRe_write/1
  Ground_32/1 -> PrkgClimaEngStReqNgcc_PrkgClimaEngStReqNgcc_write/1
  Ground_33/1 -> PrkgClimaHVReq_PrkgClimaHVReq_write/1
  Ground_34/1 -> PrkgClimaOnReqRe_PrkgClimaOnReqRe_write/1
  Ground_35/1 -> PrkgCmftRlyReq_PrkgCmftRlyReq_write/1
  Ground_36/1 -> PrkgOGMAirSrcActv_PrkgOGMAirSrcActv_write/1
  Ground_37/1 -> PrkgVntFctn_PrkgVntFctn_write/1
  Ground_38/1 -> PrvntWinFrz_PrvntWinFrz_write/1
  Ground_39/1 -> PrvntWinFrzFnshd_PrvntWinFrzFnshd_write/1
  Ground_40/1(Out1) -> RemActvSts_RemActvSts_write/1
  Ground_41/1 -> RemClimaFnshd1_RemClimaFnshd1_write/1
  Ground_42/1 -> RemClimaWarn_RemClimaWarn_write/1
  Ground_43/1 -> RemOxygenSplyActv_RemOxygenSplyActv_write/1
  Ground_44/1 -> RemOxygenSplyActvFaildNgcc_RemOxygenSplyActvFaildNgcc_write/1
  Ground_45/1 -> RemOxygenSplyFnshd_RemOxygenSplyFnshd_write/1
  Ground_46/1 -> RemOxygenSplyFnshd1_RemOxygenSplyFnshd1_write/1
  Ground_47/1 -> RemOxygenSplyWarn_RemOxygenSplyWarn_write/1
  Ground_48/1(Out1) -> RemSynchrnztnLcl_RemSynchrnztnLcl_write/1
  Ground_49/1 -> RemWinClsReq_RemWinClsReq_write/1
  Ground_50/1 -> SeatClimaDurgClimaHmiSeatHeatgForRowThrdLe_SeatClimaDurgClimaHmiSeatHeatgForRowThrdLe_write/1
  Ground_51/1 -> SeatClimaDurgClimaHmiSeatHeatgForRowThrdRi_SeatClimaDurgClimaHmiSeatHeatgForRowThrdRi_write/1
  Ground_52/1 -> SeatClimaDurgClimaHmiSeatVentnForRowThrdLe_SeatClimaDurgClimaHmiSeatVentnForRowThrdLe_write/1
  Ground_53/1 -> SeatClimaDurgClimaHmiSeatVentnForRowThrdRi_SeatClimaDurgClimaHmiSeatVentnForRowThrdRi_write/1
  Ground_54/1 -> TelmDefrostFnshd_TelmDefrostFnshd_write/1
  Ground_55/1 -> TelmSeatHeatDrvrFnshd_TelmSeatHeatDrvrFnshd_write/1
  Ground_56/1 -> TelmSeatHeatPassFnshd_TelmSeatHeatPassFnshd_write/1
  Ground_57/1 -> TelmSeatHeatSecLeFnshd_TelmSeatHeatSecLeFnshd_write/1
  Ground_58/1 -> TelmSeatHeatSecRiFnshd_TelmSeatHeatSecRiFnshd_write/1
  Ground_59/1 -> TelmSeatHeatThrdLeFnshd_TelmSeatHeatThrdLeFnshd_write/1
  Ground_60/1 -> TelmSeatHeatThrdRiFnshd_TelmSeatHeatThrdRiFnshd_write/1
  Ground_61/1 -> TelmSeatVentnDrvrFnshd_TelmSeatVentnDrvrFnshd_write/1
  Ground_62/1 -> TelmSeatVentnPassFnshd_TelmSeatVentnPassFnshd_write/1
  Ground_63/1 -> TelmSeatVentnSecLeFnshd_TelmSeatVentnSecLeFnshd_write/1
  Ground_64/1 -> TelmSeatVentnSecRiFnshd_TelmSeatVentnSecRiFnshd_write/1
  Ground_65/1 -> TelmSeatVentnThrdLeFnshd_TelmSeatVentnThrdLeFnshd_write/1
  Ground_66/1 -> TelmSeatVentnThrdRiFnshd_TelmSeatVentnThrdRiFnshd_write/1
  Ground_67/1 -> TelmSteerWhlHeatFnshd_TelmSteerWhlHeatFnshd_write/1
  Ground_68/1 -> VMMChngNdMtnNgcc_VMMChngNdMtnNgcc_write/1
  Ground_69/1 -> VTMOverTProtection_VTMOverTProtection_write/1
  Ground_70/1 -> VTMOverTProtectionFnshd_VTMOverTProtectionFnshd_write/1
  Ground_71/1 -> BlwAftRunReq_BlwAftRunReq_write/1
  Ground_72/1 -> BlwAftRunSts_BlwAftRunSts_write/1
  Ground_73/1 -> BookTrvlClimaActv_BookTrvlClimaActv_write/1
  Ground_74/1 -> CdsParkgClimaActv_CdsParkgClimaActv_write/1
  Ground_75/1 -> ClimaActv_Ngcc_ClimaActv_write/1
  Ground_76/1 -> ClimaOvrHeatProActv_ClimaOvrHeatProActv_write/1
  Ground_77/1 -> ClimaOvrHeatProActvSts_ClimaOvrHeatProActvSts_write/1
  Ground_78/1 -> ClimaOvrHeatProWarn_ClimaOvrHeatProWarn_write/1
  Ground_79/1 -> ClimaOvrHeatPrtSts_ClimaOvrHeatPrtSts_write/1
  Ground_80/1 -> ClimaSts_ClimaSts_write/1
  Ground_81/1 -> ClimaTiOfEnd_Ngcc_ClimaTiOfEnd_write/1
  Ground_82/1 -> ClimaWarn_ClimaWarn_write/1
  Ground_83/1 -> ClngActv_ClngActv_write/1
  Ground_84/1 -> Co2WarnForTelm_Co2WarnForTelm_write/1
  Ground_85/1 -> COHSts_COHSts_write/1
  Ground_86/1 -> CoolgFastForPrePrkgClimaActv_CoolgFastForPrePrkgClimaActv_write/1
  Ground_87/1 -> CoolgFastUniqueForPrePrkgClimaActv_CoolgFastUniqueForPrePrkgClimaActv_write/1
  Ground_88/1(Out1) -> DefrstReq_DefrstReq_write/1
  Ground_89/1 -> DiDblLock_DiDblLock_write/1
  Ground_90/1 -> EcuKeepActvFromPrkgClima_EcuKeepActvFromPrkgClima_write/1
  Ground_91/1 -> ExtVentFanVolStsFrmClima_ExtVentFanVolStsFrmClima_write/1
  Ground_92/1 -> ExtVentStsFrmClima_ExtVentStsFrmClima_write/1
  Ground_93/1(Out1) -> ExtVentTempStsFrmClima_ExtVentTempStsFrmClima_write/1
  Ground_94/1 -> ExtVentTempStsFrmClimaExtVentTemprSts_ExtVentTempStsFrmClimaExtVentTemprSts_write/1
  Ground_95/1 -> ExtVentTempStsFrmClimaExtVentTemprVal_ExtVentTempStsFrmClimaExtVentTemprVal_write/1
  Ground_96/1 -> ExtVentToastFrmClima_Prkg_ExtVentToastFrmClima_write/1
  Ground_97/1 -> HeatrElecFrntReqFromPrkgClima_HeatrElecFrntReqFromPrkgClima_write/1
  Ground_98/1 -> IntCo2ActvSts_IntCo2ActvSts_write/1
  Ground_99/1 -> KeyClimaActv_KeyClimaActv_write/1
  Ground_100/1 -> MemSaveFlgForPrkgClimaActvMgr_MemSaveFlgForPrkgClimaActvMgr_write/1
  Ground_101/1(Out1) -> MemWrForPrkgClimaActvMgr_MemWrForPrkgClimaActvMgr_write/1
  Ground_102/1 -> ModForPrkgClima_ModForPrkgClima_write/1
  Ground_103/1 -> PreClngActv_PreClngActv_write/1
  Ground_104/1 -> PreClngNotif_PreClngNotif_write/1
  Ground_105/1 -> PreClngSts_PreClngSts_write/1
  Ground_106/1 -> PrkgClimaActvMgrVFCBodyPreClimatisation_PrkgClimaActvMgrVFCBodyPreClimatisation_write/1
  Ground_107/1 -> PrkgClimaActvMgrVFCGlobalShortDuration_PrkgClimaActvMgrVFCGlobalShortDuration_write/1
  Ground_108/1 -> PrkgClimaActvMgrVFCLocking_PrkgClimaActvMgrVFCLocking_write/1
  Ground_109/1 -> PrkgClimaActvMgrVFCParkingDrivingClimatization_PrkgClimaActvMgrVFCParkingDrivingClimatization_write/1
  Ground_110/1 -> PrkgClimaActvMgrVFCPostClimatisation_PrkgClimaActvMgrVFCPostClimatisation_write/1
  Ground_111/1 -> PrkgClimaActvMgrVFCWindows_PrkgClimaActvMgrVFCWindows_write/1
  Ground_112/1 -> PrkgClimaAirflowReq_PrkgClimaAirflowReq_write/1
  Ground_113/1 -> PrkgClimaFnshd_PrkgClimaFnshd_write/1
  Ground_114/1 -> PrkgClimaHeatgReq_PrkgClimaHeatgReq_write/1
  Ground_115/1 -> PrkgClimaModReq_PrkgClimaModReq_write/1
  Ground_116/1 -> PrkgClimaOnReq_PrkgClimaOnReq_write/1
  Ground_117/1 -> PrkgClimaPostCdngReq_PrkgClimaPostCdngReq_write/1
  Ground_118/1 -> PrkgClimaPreCdngReq_PrkgClimaPreCdngReq_write/1
  Ground_119/1 -> PrkgClimaRecirReq_PrkgClimaRecirReq_write/1
  Ground_120/1 -> PrkgClimaRqrdImdtForBattULo_PrkgClimaRqrdImdtForBattULo_write/1
  Ground_121/1 -> PrkgClimaRqrdImdtFromActvMgr_PrkgClimaRqrdImdtFromActvMgr_write/1
  Ground_122/1 -> PrkgClimaRqrdTmrForBattULo_PrkgClimaRqrdTmrForBattULo_write/1
  Ground_123/1 -> PrkgClimaTemp_PrkgClimaTemp_write/1
  Ground_124/1 -> PrkgClimaWarn_PrkgClimaWarn_write/1
  Ground_125/1 -> PrkgModMgr_PrkgModMgr_write/1
  Ground_126/1 -> RemClimaActv_RemClimaActv_write/1
  Ground_127/1 -> RemClimaDefrstSts_RemClimaDefrstSts_write/1
  Ground_128/1 -> RemClimaDelaySts_RemClimaDelaySts_write/1
  Ground_129/1 -> RemClimaEgyFinalReq_RemClimaEgyFinalReq_write/1
  Ground_130/1 -> RemClimaExtnTiRspn_RemClimaExtnTiRspn_write/1
  Ground_131/1 -> RemClimaFnshd_RemClimaFnshd_write/1
  Ground_132/1 -> RemClimaHvSts_RemClimaHvSts_write/1
  Ground_133/1 -> RemStrtClimaRspn_RemStrtClimaRspn_write/1
  Ground_134/1 -> RemVentActvSts_RemVentActvSts_write/1
  Ground_135/1 -> RemVentFbSts_RemVentFbSts_write/1
  Ground_136/1 -> RemVentReqRspnFb_RemVentReqRspnFb_write/1
  Ground_137/1 -> RemVentWarnSts_RemVentWarnSts_write/1
  Ground_138/1 -> ReqForPrkgClima_ReqForPrkgClima_write/1
  Ground_139/1 -> ReqForSwtHeatrTypFromActvMgr_ReqForSwtHeatrTypFromActvMgr_write/1
  Ground_140/1 -> RoofOpnReqFrmClima_RoofOpnReqFrmClima_write/1
  Ground_141/1 -> RtLeForPrkgClima_RtLeForPrkgClima_write/1
  Ground_142/1 -> SchedCmptHeatCoolFB_SchedCmptHeatCoolFB_write/1
  Ground_143/1(Out1) -> SeatClimaDurgClima_SeatClimaDurgClima_write/1
  Ground_144/1 -> SteerWhlHeatgDurgClima_Ngcc_SteerWhlHeatgDurgClima_write/1
  Ground_145/1 -> SuspStrtActvForPrkgClima_SuspStrtActvForPrkgClima_write/1
  Ground_146/1 -> WarnForHybWoUFromActvMgr_WarnForHybWoUFromActvMgr_write/1
